simulink model "AcasXuClosedLoop_all_comp2017b"  (Simulink 9.0)
settings: Solver=VariableStepAuto  StartTime=0.0  StopTime=20  RelTol=1e-3
libraries: neural x14040, simulink x2
(part 25 of 534)

== system AcasXuClosedLoop_all_comp2017b/networks/Networks_m_6/AcasXu_1_6/Layer 5 ==
  "a{4} " : Inport  PortDimensions=50
      mask Display: e = 0.06;c1 = [120 230 180]/255;c2 = ([120 230 180]+0)/(256+128);c3 = ([120 230 180]+128)/(256+128);patch([0 1 1 0],[0 0 1 1],c1);patch([0 1 1 1-e 1-e 0+e],[0 0 1 1-e 0+e 0+e],c2);patch([0 0 1 1-e 0+e 0+e],[0 1 1 1-e 1-e 0+e],c3);plot([0 1 1 0 0],[0 0 1 1 0]);
  Delays 1 : Subsystem
      mask Display: e = 0.06;c1 = [120 150 230]/255;c2 = ([120 150 230]+0)/383;c3 = ([120 150 230]+128)/383;patch([0 1 1 0],[0 0 1 1],c1);patch([0 1 1 1-e 1-e 0+e],[0 0 1 1-e 0+e 0+e],c2);patch([0 0 1 1-e 0+e 0+e],[0 1 1 1-e 1-e 0+e],c3);plot([0 1 1 0 0],[0 0 1 1 0]);disp('0');
  "LW{5,4}" : Subsystem
      mask Display: e = 0.06;c1 = [120 150 230]/255;c2 = ([120 150 230]+0)/383;c3 = ([120 150 230]+128)/383;patch([0 1 1 0],[0 0 1 1],c1);patch([0 1 1 1-e 1-e 0+e],[0 0 1 1-e 0+e 0+e],c2);patch([0 0 1 1-e 0+e 0+e],[0 1 1 1-e 1-e 0+e],c3);plot([0 1 1 0 0],[0 0 1 1 0]);disp('W');
  "b{5}" : Constant  Value=[0.3707949999999999857180910112219862639904022216796875;-0.152619000000000004657607632907456718385219573974609375;0.048162000000000003419042826635632081888616085052490234375;0.0157031000000000010852208021105980151332914829254150390625;-0.5211959999999999926245664028101600706577301025390625;0.98311000000000003939959469789755530655384063720703125;-0.299582999999999988194332445345935411751270294189453125;-0.1225920000000000065210059574383194558322429656982421875;-0.02888759999999999938946615429813391529023647308349609375;0.281310000000000004494182803682633675634860992431640625;0.2471440000000000025703883466121624223887920379638671875;0.291130999999999973137931874589412473142147064208984375;0.12004800000000000193001170600837212987244129180908203125;-0.25239000000000000323296944770845584571361541748046875;-0.0200420000000000007867040352493859245441854000091552734375;0.06623010000000000008224532166423159651458263397216796875;0.175796000000000007812417379682301543653011322021484375;-0.12461899999999999366639968911840696819126605987548828125;0.17621100000000000651567688692011870443820953369140625;-0.450682000000000027029045668314211070537567138671875;-0.048770700000000000162714286489062942564487457275390625;0.13494200000000000638777919448330067098140716552734375;-1.5442299999999999915445414444548077881336212158203125;-0.032870799999999998630695330348316929303109645843505859375;0.153853999999999990766497148797498084604740142822265625;-0.86296600000000001084998757505672983825206756591796875;-0.5057430000000000536175548404571600258350372314453125;-0.51248400000000005061195906819193623960018157958984375;-0.269037000000000026123103680220083333551883697509765625;0.07316989999999999605417855264022364281117916107177734375;0.1014589999999999936353134444289025850594043731689453125;0.1659440000000000081659123907229513861238956451416015625;-0.63569200000000003480948862488730810582637786865234375;-0.2168150000000000077182704671940882690250873565673828125;0.158696000000000003726796649061725474894046783447265625;-1.9692700000000000759570184527547098696231842041015625;-0.76643799999999995264943208894692361354827880859375;0.299881000000000008665068662594421766698360443115234375;-0.60654200000000002557243306000600568950176239013671875;0.1070440000000000002611244553918368183076381683349609375;-0.0182207999999999988027798991652161930687725543975830078125;0.1372770000000000101270103414208278991281986236572265625;-0.08875829999999999830873065320702153258025646209716796875;0.1260400000000000131361588273648521862924098968505859375;0.07690940000000000276170197821556939743459224700927734375;0.5071440000000000392077481592423282563686370849609375;0.251952000000000009283240842705708928406238555908203125;-0.1578380000000000060733640339094563387334346771240234375;-2.0016400000000000858335624798201024532318115234375;0.03576759999999999661834948483374319039285182952880859375]
      mask Display: e = 0.06;c1 = [120 150 230]/255;c2 = ([120 150 230]+0)/383;c3 = ([120 150 230]+128)/383;patch([0 1 1 0],[0 0 1 1],c1);patch([0 1 1 1-e 1-e 0+e],[0 0 1 1-e 0+e 0+e],c2);patch([0 0 1 1-e 0+e 0+e],[0 1 1 1-e 1-e 0+e],c3);plot([0 1 1 0 0],[0 0 1 1 0]);disp('b');
  netsum : Sum  Inputs=2  SaturateOnIntegerOverflow=on  InputSameDT=on  OutDataTypeStr=Inherit: Same as first input
      mask Display: e = 0.06;c1 = [120 150 230]/255;c2 = ([120 150 230]+0)/383;c3 = ([120 150 230]+128)/383;patch([0 1 1 0],[0 0 1 1],c1);patch([0 1 1 1-e 1-e 0+e],[0 0 1 1-e 0+e 0+e],c2);patch([0 0 1 1-e 0+e 0+e],[0 1 1 1-e 1-e 0+e],c3);plot([0 1 1 0 0],[0 0 1 1 0]);disp('+');
  poslin : neural/Transfer Functions/poslin
      settings: SourceProductName=Deep Learning Toolbox  SourceProductBaseCode=NN
  "a{5}" : Outport  InitialOutput=[0;0;0;0;0;0;0;0;0;0;0;0;0;0;0;0;0;0;0;0;0;0;0;0;0;0;0;0;0;0;0;0;0;0;0;0;0;0;0;0;0;0;0;0;0;0;0;0;0;0]
      mask Display: e = 0.06;c1 = [120 230 180]/255;c2 = ([120 230 180]+0)/(256+128);c3 = ([120 230 180]+128)/(256+128);patch([0 1 1 0],[0 0 1 1],c1);patch([0 1 1 1-e 1-e 0+e],[0 0 1 1-e 0+e 0+e],c2);patch([0 0 1 1-e 0+e 0+e],[0 1 1 1-e 1-e 0+e],c3);plot([0 1 1 0 0],[0 0 1 1 0]);
  wires:
  poslin/1 -> "a{5}"/1
  netsum/1 -> poslin/1
  "b{5}"/1 -> netsum/2
  "LW{5,4}"/1("lz{5,4}") -> netsum/1
  Delays 1/1("pd{5,4}") -> "LW{5,4}"/1("ad{5,4}")
  "a{4} "/1 -> Delays 1/1("p{5}")

== system AcasXuClosedLoop_all_comp2017b/networks/Networks_m_6/AcasXu_1_6/Layer 6 ==
  "a{5} " : Inport  PortDimensions=50
      mask Display: e = 0.06;c1 = [120 230 180]/255;c2 = ([120 230 180]+0)/(256+128);c3 = ([120 230 180]+128)/(256+128);patch([0 1 1 0],[0 0 1 1],c1);patch([0 1 1 1-e 1-e 0+e],[0 0 1 1-e 0+e 0+e],c2);patch([0 0 1 1-e 0+e 0+e],[0 1 1 1-e 1-e 0+e],c3);plot([0 1 1 0 0],[0 0 1 1 0]);
  Delays 1 : Subsystem
      mask Display: e = 0.06;c1 = [120 150 230]/255;c2 = ([120 150 230]+0)/383;c3 = ([120 150 230]+128)/383;patch([0 1 1 0],[0 0 1 1],c1);patch([0 1 1 1-e 1-e 0+e],[0 0 1 1-e 0+e 0+e],c2);patch([0 0 1 1-e 0+e 0+e],[0 1 1 1-e 1-e 0+e],c3);plot([0 1 1 0 0],[0 0 1 1 0]);disp('0');
  "LW{6,5}" : Subsystem
      mask Display: e = 0.06;c1 = [120 150 230]/255;c2 = ([120 150 230]+0)/383;c3 = ([120 150 230]+128)/383;patch([0 1 1 0],[0 0 1 1],c1);patch([0 1 1 1-e 1-e 0+e],[0 0 1 1-e 0+e 0+e],c2);patch([0 0 1 1-e 0+e 0+e],[0 1 1 1-e 1-e 0+e],c3);plot([0 1 1 0 0],[0 0 1 1 0]);disp('W');
  "b{6}" : Constant  Value=[-0.09293780000000000096616048494979622773826122283935546875;-0.0196711000000000003906652779051000834442675113677978515625;-0.08280949999999999422417573669008561410009860992431640625;-0.054471199999999997398703044382273219525814056396484375;-0.03268479999999999996429522752805496565997600555419921875;0.11851000000000000422772927777259610593318939208984375;-0.13510400000000000186872739504906348884105682373046875;-0.1563849999999999962341235004714690148830413818359375;-0.660301000000000026801671992870979011058807373046875;-0.04415539999999999742907874633601750247180461883544921875;0.03159220000000000083684170704145799390971660614013671875;-0.03956199999999999994404475955889211036264896392822265625;-0.80943799999999999084110413605230860412120819091796875;0.1153389999999999970814457128653884865343570709228515625;-0.1059650000000000036326497365735122002661228179931640625;-0.39901500000000000856203996590920723974704742431640625;-0.10792300000000000503863617495881044305860996246337890625;-0.293030999999999985927701118271215818822383880615234375;0.1441389999999999893542934614742989651858806610107421875;-0.06593970000000000386553011821888503618538379669189453125;-0.9216029999999999500204239666345529258251190185546875;-0.1278370000000000061834981579522718675434589385986328125;-0.03856020000000000280682144193633575923740863800048828125;0.026769199999999999939337413934481446631252765655517578125;-0.0158453999999999990688781537073737126775085926055908203125;0.0284409000000000015961898469640800612978637218475341796875;-0.03193300000000000304911651483052992261946201324462890625;0.1266130000000000033200109328390681184828281402587890625;-0.95733100000000004303757350498926825821399688720703125;-0.335019000000000011230127938688383437693119049072265625;-0.271828000000000014058088027013582177460193634033203125;0.06131860000000000088249407781404443085193634033203125;-0.59949500000000000010658141036401502788066864013671875;0.08274660000000000348308049069601111114025115966796875;-0.280430000000000012594369991347775794565677642822265625;-0.1293509999999999937614347800263203680515289306640625;0.08134900000000000463984406451345421373844146728515625;-0.07751650000000000206856753948159166611731052398681640625;-2.159569999999999989626076057902537286281585693359375;0.046974099999999997800959050664459937252104282379150390625;0.07693919999999999925766047681463533081114292144775390625;0.07165760000000000184972037686748080886900424957275390625;-0.48705100000000001170263885796885006129741668701171875;-0.23598500000000000031974423109204508364200592041015625;-1.21154999999999990478727340814657509326934814453125;0.04766320000000000278728151670293300412595272064208984375;-0.148309999999999997388755446081631816923618316650390625;-0.9624960000000000182041048901737667620182037353515625;-0.052430699999999996752109154840582050383090972900390625;-0.9180289999999999839275233171065337955951690673828125]
      mask Display: e = 0.06;c1 = [120 150 230]/255;c2 = ([120 150 230]+0)/383;c3 = ([120 150 230]+128)/383;patch([0 1 1 0],[0 0 1 1],c1);patch([0 1 1 1-e 1-e 0+e],[0 0 1 1-e 0+e 0+e],c2);patch([0 0 1 1-e 0+e 0+e],[0 1 1 1-e 1-e 0+e],c3);plot([0 1 1 0 0],[0 0 1 1 0]);disp('b');
  netsum : Sum  Inputs=2  SaturateOnIntegerOverflow=on  InputSameDT=on  OutDataTypeStr=Inherit: Same as first input
      mask Display: e = 0.06;c1 = [120 150 230]/255;c2 = ([120 150 230]+0)/383;c3 = ([120 150 230]+128)/383;patch([0 1 1 0],[0 0 1 1],c1);patch([0 1 1 1-e 1-e 0+e],[0 0 1 1-e 0+e 0+e],c2);patch([0 0 1 1-e 0+e 0+e],[0 1 1 1-e 1-e 0+e],c3);plot([0 1 1 0 0],[0 0 1 1 0]);disp('+');
  poslin : neural/Transfer Functions/poslin
      settings: SourceProductName=Deep Learning Toolbox  SourceProductBaseCode=NN
  "a{6}" : Outport  InitialOutput=[0;0;0;0;0;0;0;0;0;0;0;0;0;0;0;0;0;0;0;0;0;0;0;0;0;0;0;0;0;0;0;0;0;0;0;0;0;0;0;0;0;0;0;0;0;0;0;0;0;0]
      mask Display: e = 0.06;c1 = [120 230 180]/255;c2 = ([120 230 180]+0)/(256+128);c3 = ([120 230 180]+128)/(256+128);patch([0 1 1 0],[0 0 1 1],c1);patch([0 1 1 1-e 1-e 0+e],[0 0 1 1-e 0+e 0+e],c2);patch([0 0 1 1-e 0+e 0+e],[0 1 1 1-e 1-e 0+e],c3);plot([0 1 1 0 0],[0 0 1 1 0]);
  wires:
  poslin/1 -> "a{6}"/1
  netsum/1 -> poslin/1
  "b{6}"/1 -> netsum/2
  "LW{6,5}"/1("lz{6,5}") -> netsum/1
  Delays 1/1("pd{6,5}") -> "LW{6,5}"/1("ad{6,5}")
  "a{5} "/1 -> Delays 1/1("p{6}")

== system AcasXuClosedLoop_all_comp2017b/networks/Networks_m_6/AcasXu_1_6/Layer 7 ==
  "a{6} " : Inport  PortDimensions=50
      mask Display: e = 0.06;c1 = [120 230 180]/255;c2 = ([120 230 180]+0)/(256+128);c3 = ([120 230 180]+128)/(256+128);patch([0 1 1 0],[0 0 1 1],c1);patch([0 1 1 1-e 1-e 0+e],[0 0 1 1-e 0+e 0+e],c2);patch([0 0 1 1-e 0+e 0+e],[0 1 1 1-e 1-e 0+e],c3);plot([0 1 1 0 0],[0 0 1 1 0]);
  Delays 1 : Subsystem
      mask Display: e = 0.06;c1 = [120 150 230]/255;c2 = ([120 150 230]+0)/383;c3 = ([120 150 230]+128)/383;patch([0 1 1 0],[0 0 1 1],c1);patch([0 1 1 1-e 1-e 0+e],[0 0 1 1-e 0+e 0+e],c2);patch([0 0 1 1-e 0+e 0+e],[0 1 1 1-e 1-e 0+e],c3);plot([0 1 1 0 0],[0 0 1 1 0]);disp('0');
  "LW{7,6}" : Subsystem
      mask Display: e = 0.06;c1 = [120 150 230]/255;c2 = ([120 150 230]+0)/383;c3 = ([120 150 230]+128)/383;patch([0 1 1 0],[0 0 1 1],c1);patch([0 1 1 1-e 1-e 0+e],[0 0 1 1-e 0+e 0+e],c2);patch([0 0 1 1-e 0+e 0+e],[0 1 1 1-e 1-e 0+e],c3);plot([0 1 1 0 0],[0 0 1 1 0]);disp('W');
  "b{7}" : Constant  Value=[-0.0201359000000000017138734875743466545827686786651611328125;-0.01936569999999999958095742158548091538250446319580078125;-0.0194450000000000004785061236134424689225852489471435546875;-0.01923740000000000172075687032702262513339519500732421875;-0.01934299999999999908339987086947076022624969482421875]
      mask Display: e = 0.06;c1 = [120 150 230]/255;c2 = ([120 150 230]+0)/383;c3 = ([120 150 230]+128)/383;patch([0 1 1 0],[0 0 1 1],c1);patch([0 1 1 1-e 1-e 0+e],[0 0 1 1-e 0+e 0+e],c2);patch([0 0 1 1-e 0+e 0+e],[0 1 1 1-e 1-e 0+e],c3);plot([0 1 1 0 0],[0 0 1 1 0]);disp('b');
  netsum : Sum  Inputs=2  SaturateOnIntegerOverflow=on  InputSameDT=on  OutDataTypeStr=Inherit: Same as first input
      mask Display: e = 0.06;c1 = [120 150 230]/255;c2 = ([120 150 230]+0)/383;c3 = ([120 150 230]+128)/383;patch([0 1 1 0],[0 0 1 1],c1);patch([0 1 1 1-e 1-e 0+e],[0 0 1 1-e 0+e 0+e],c2);patch([0 0 1 1-e 0+e 0+e],[0 1 1 1-e 1-e 0+e],c3);plot([0 1 1 0 0],[0 0 1 1 0]);disp('+');
  purelin : neural/Transfer Functions/purelin
      settings: SourceProductName=Deep Learning Toolbox
  "a{7}" : Outport  InitialOutput=[0;0;0;0;0]
      mask Display: e = 0.06;c1 = [120 230 180]/255;c2 = ([120 230 180]+0)/(256+128);c3 = ([120 230 180]+128)/(256+128);patch([0 1 1 0],[0 0 1 1],c1);patch([0 1 1 1-e 1-e 0+e],[0 0 1 1-e 0+e 0+e],c2);patch([0 0 1 1-e 0+e 0+e],[0 1 1 1-e 1-e 0+e],c3);plot([0 1 1 0 0],[0 0 1 1 0]);
  wires:
  purelin/1 -> "a{7}"/1
  netsum/1 -> purelin/1
  "b{7}"/1 -> netsum/2
  "LW{7,6}"/1("lz{7,6}") -> netsum/1
  Delays 1/1("pd{7,6}") -> "LW{7,6}"/1("ad{7,6}")
  "a{6} "/1 -> Delays 1/1("p{7}")

== system AcasXuClosedLoop_all_comp2017b/networks/Networks_m_6/AcasXu_1_6/Process Input 1 ==
  x : Inport  PortDimensions=5
      mask Display: e = 0.06;c1 = [120 230 180]/255;c2 = ([120 230 180]+0)/(256+128);c3 = ([120 230 180]+128)/(256+128);patch([0 1 1 0],[0 0 1 1],c1);patch([0 1 1 1-e 1-e 0+e],[0 0 1 1-e 0+e 0+e],c2);patch([0 0 1 1-e 0+e 0+e],[0 1 1 1-e 1-e 0+e],c3);plot([0 1 1 0 0],[0 0 1 1 0]);
  p : Outport  PortDimensions=5
      mask Display: e = 0.06;c1 = [120 230 180]/255;c2 = ([120 230 180]+0)/(256+128);c3 = ([120 230 180]+128)/(256+128);patch([0 1 1 0],[0 0 1 1],c1);patch([0 1 1 1-e 1-e 0+e],[0 0 1 1-e 0+e 0+e],c2);patch([0 0 1 1-e 0+e 0+e],[0 1 1 1-e 1-e 0+e],c3);plot([0 1 1 0 0],[0 0 1 1 0]);
  wires:
  x/1 -> p/1

== system AcasXuClosedLoop_all_comp2017b/networks/Networks_m_6/AcasXu_1_6/Process Output 1 ==
  a : Inport  PortDimensions=5
      mask Display: e = 0.06;c1 = [120 230 180]/255;c2 = ([120 230 180]+0)/(256+128);c3 = ([120 230 180]+128)/(256+128);patch([0 1 1 0],[0 0 1 1],c1);patch([0 1 1 1-e 1-e 0+e],[0 0 1 1-e 0+e 0+e],c2);patch([0 0 1 1-e 0+e 0+e],[0 1 1 1-e 1-e 0+e],c3);plot([0 1 1 0 0],[0 0 1 1 0]);
  y : Outport  PortDimensions=5
      mask Display: e = 0.06;c1 = [120 230 180]/255;c2 = ([120 230 180]+0)/(256+128);c3 = ([120 230 180]+128)/(256+128);patch([0 1 1 0],[0 0 1 1],c1);patch([0 1 1 1-e 1-e 0+e],[0 0 1 1-e 0+e 0+e],c2);patch([0 0 1 1-e 0+e 0+e],[0 1 1 1-e 1-e 0+e],c3);plot([0 1 1 0 0],[0 0 1 1 0]);
  wires:
  a/1 -> y/1

== system AcasXuClosedLoop_all_comp2017b/networks/Networks_m_6/AcasXu_2_6/Layer 1 ==
  "p{1}" : Inport  PortDimensions=5
      mask Display: e = 0.06;c1 = [120 230 180]/255;c2 = ([120 230 180]+0)/(256+128);c3 = ([120 230 180]+128)/(256+128);patch([0 1 1 0],[0 0 1 1],c1);patch([0 1 1 1-e 1-e 0+e],[0 0 1 1-e 0+e 0+e],c2);patch([0 0 1 1-e 0+e 0+e],[0 1 1 1-e 1-e 0+e],c3);plot([0 1 1 0 0],[0 0 1 1 0]);
  Delays 1 : Subsystem
      mask Display: e = 0.06;c1 = [120 150 230]/255;c2 = ([120 150 230]+0)/383;c3 = ([120 150 230]+128)/383;patch([0 1 1 0],[0 0 1 1],c1);patch([0 1 1 1-e 1-e 0+e],[0 0 1 1-e 0+e 0+e],c2);patch([0 0 1 1-e 0+e 0+e],[0 1 1 1-e 1-e 0+e],c3);plot([0 1 1 0 0],[0 0 1 1 0]);disp('0');
  "IW{1,1}" : Subsystem
      mask Display: e = 0.06;c1 = [120 150 230]/255;c2 = ([120 150 230]+0)/383;c3 = ([120 150 230]+128)/383;patch([0 1 1 0],[0 0 1 1],c1);patch([0 1 1 1-e 1-e 0+e],[0 0 1 1-e 0+e 0+e],c2);patch([0 0 1 1-e 0+e 0+e],[0 1 1 1-e 1-e 0+e],c3);plot([0 1 1 0 0],[0 0 1 1 0]);disp('W');
  "b{1}" : Constant  Value=[-0.06689219999999999888284918370118248276412487030029296875;-0.409326000000000023160140472100465558469295501708984375;0.0319440000000000001723066134218242950737476348876953125;0.01255019999999999920359261551539020729251205921173095703125;-0.459266000000000007563727422166266478598117828369140625;-0.0239383999999999985686116588112781755626201629638671875;-0.022123099999999999709654474600029061548411846160888671875;-0.377510999999999985465848340027150698006153106689453125;0.199315999999999993175237023024237714707851409912109375;0.065776200000000006884448566779610700905323028564453125;-0.25278699999999998393462874446413479745388031005859375;-0.06722430000000000072102324111256166361272335052490234375;0.52313500000000001666222715357434935867786407470703125;-0.273511000000000004117595153729780577123165130615234375;-0.112341999999999997417177155512035824358463287353515625;-0.2958819999999999783568682687473483383655548095703125;0.084172899999999994946620063274167478084564208984375;0.01048840000000000015345502646368913701735436916351318359375;-0.7274350000000000537880850970395840704441070556640625;0.03777819999999999789341842415524297393858432769775390625;0.08883770000000000555129275880972272716462612152099609375;-0.171831000000000011507239605634822510182857513427734375;-0.01849019999999999830375685405670083127915859222412109375;-0.2042090000000000016289192217300296761095523834228515625;-0.00886104000000000058268057046007015742361545562744140625;-0.398774000000000017340795466225245036184787750244140625;0.252219000000000026506796757530537433922290802001953125;0.1525330000000000019166890297128702513873577117919921875;-0.416878000000000026314950218875310383737087249755859375;0.018960600000000001064126564642720040865242481231689453125;0.340818000000000009830358749240986071527004241943359375;-0.09769619999999999693240937403970747254788875579833984375;0.031457199999999997663824302662760601378977298736572265625;-0.376325000000000020605739337042905390262603759765625;-0.1449499999999999955146989805143675766885280609130859375;-0.389840000000000019841905896100797690451145172119140625;0.016368500000000001104449864897105726413428783416748046875;-0.34057999999999999385380533567513339221477508544921875;-0.1790029999999999954507501342959585599601268768310546875;-0.0213322999999999984854781587273464538156986236572265625;0.19489699999999998691890823465655557811260223388671875;-0.07400089999999999446078646769819897599518299102783203125;-0.1605999999999999927613458794439793564379215240478515625;-0.362099000000000004195754854663391597568988800048828125;0.18424099999999998811262003073352389037609100341796875;-0.2050929999999999975290876363942516036331653594970703125;-0.0325461999999999973542941233972669579088687896728515625;-0.12970599999999998797051148358150385320186614990234375;0.04095300000000000328892468814956373535096645355224609375;-0.409160000000000023678836669205338694155216217041015625]
      mask Display: e = 0.06;c1 = [120 150 230]/255;c2 = ([120 150 230]+0)/383;c3 = ([120 150 230]+128)/383;patch([0 1 1 0],[0 0 1 1],c1);patch([0 1 1 1-e 1-e 0+e],[0 0 1 1-e 0+e 0+e],c2);patch([0 0 1 1-e 0+e 0+e],[0 1 1 1-e 1-e 0+e],c3);plot([0 1 1 0 0],[0 0 1 1 0]);disp('b');
  netsum : Sum  Inputs=2  SaturateOnIntegerOverflow=on  InputSameDT=on  OutDataTypeStr=Inherit: Same as first input
      mask Display: e = 0.06;c1 = [120 150 230]/255;c2 = ([120 150 230]+0)/383;c3 = ([120 150 230]+128)/383;patch([0 1 1 0],[0 0 1 1],c1);patch([0 1 1 1-e 1-e 0+e],[0 0 1 1-e 0+e 0+e],c2);patch([0 0 1 1-e 0+e 0+e],[0 1 1 1-e 1-e 0+e],c3);plot([0 1 1 0 0],[0 0 1 1 0]);disp('+');
  poslin : neural/Transfer Functions/poslin
      settings: SourceProductName=Deep Learning Toolbox  SourceProductBaseCode=NN
  "a{1}" : Outport  InitialOutput=[0;0;0;0;0;0;0;0;0;0;0;0;0;0;0;0;0;0;0;0;0;0;0;0;0;0;0;0;0;0;0;0;0;0;0;0;0;0;0;0;0;0;0;0;0;0;0;0;0;0]
      mask Display: e = 0.06;c1 = [120 230 180]/255;c2 = ([120 230 180]+0)/(256+128);c3 = ([120 230 180]+128)/(256+128);patch([0 1 1 0],[0 0 1 1],c1);patch([0 1 1 1-e 1-e 0+e],[0 0 1 1-e 0+e 0+e],c2);patch([0 0 1 1-e 0+e 0+e],[0 1 1 1-e 1-e 0+e],c3);plot([0 1 1 0 0],[0 0 1 1 0]);
  wires:
  poslin/1 -> "a{1}"/1
  netsum/1 -> poslin/1
  "b{1}"/1 -> netsum/2
  "IW{1,1}"/1("iz{1,1}") -> netsum/1
  Delays 1/1("pd{1,1}") -> "IW{1,1}"/1("pd{1,1}")
  "p{1}"/1 -> Delays 1/1("p{1}")

== system AcasXuClosedLoop_all_comp2017b/networks/Networks_m_6/AcasXu_2_6/Layer 2 ==
  "a{1} " : Inport  PortDimensions=50
      mask Display: e = 0.06;c1 = [120 230 180]/255;c2 = ([120 230 180]+0)/(256+128);c3 = ([120 230 180]+128)/(256+128);patch([0 1 1 0],[0 0 1 1],c1);patch([0 1 1 1-e 1-e 0+e],[0 0 1 1-e 0+e 0+e],c2);patch([0 0 1 1-e 0+e 0+e],[0 1 1 1-e 1-e 0+e],c3);plot([0 1 1 0 0],[0 0 1 1 0]);
  Delays 1 : Subsystem
      mask Display: e = 0.06;c1 = [120 150 230]/255;c2 = ([120 150 230]+0)/383;c3 = ([120 150 230]+128)/383;patch([0 1 1 0],[0 0 1 1],c1);patch([0 1 1 1-e 1-e 0+e],[0 0 1 1-e 0+e 0+e],c2);patch([0 0 1 1-e 0+e 0+e],[0 1 1 1-e 1-e 0+e],c3);plot([0 1 1 0 0],[0 0 1 1 0]);disp('0');
  "LW{2,1}" : Subsystem
      mask Display: e = 0.06;c1 = [120 150 230]/255;c2 = ([120 150 230]+0)/383;c3 = ([120 150 230]+128)/383;patch([0 1 1 0],[0 0 1 1],c1);patch([0 1 1 1-e 1-e 0+e],[0 0 1 1-e 0+e 0+e],c2);patch([0 0 1 1-e 0+e 0+e],[0 1 1 1-e 1-e 0+e],c3);plot([0 1 1 0 0],[0 0 1 1 0]);disp('W');
  "b{2}" : Constant  Value=[-0.0267394999999999993800514630493125878274440765380859375;1.202420000000000044337866711430251598358154296875;0.0700510000000000021547208461925038136541843414306640625;-0.00306441999999999999115818383188525331206619739532470703125;1.192660000000000053432813729159533977508544921875;-1.0100899999999999323563315556384623050689697265625;-0.8294289999999999718482968091848306357860565185546875;-0.032258399999999999574473719121670001186430454254150390625;-0.3208860000000000045616843635798431932926177978515625;0.88836499999999996024513393422239460051059722900390625;-0.0128079000000000005343725462125803460367023944854736328125;-0.57480799999999998561861502821557223796844482421875;-0.015656599999999999683897300428725429810583591461181640625;0.382274999999999975930364826126606203615665435791015625;1.26560000000000005826450433232821524143218994140625;-0.341955000000000008952838470577262341976165771484375;-0.06256899999999999961719510110924602486193180084228515625;-1.0951500000000000678568312650895677506923675537109375;1.0387100000000000221689333557151257991790771484375;0.6711549999999999460698063558083958923816680908203125;0.032979899999999999493649482928958605043590068817138671875;-0.265936000000000005716316309190005995333194732666015625;-1.6442200000000000148503431773860938847064971923828125;0.405764999999999986801668683256139047443866729736328125;-0.015960900000000000087396756498492322862148284912109375;0.4590520000000000155893076225765980780124664306640625;1.266480000000000050164317144663073122501373291015625;-0.006008389999999999737945177713527300511486828327178955078125;0.276619000000000003769429213207331486046314239501953125;0.12648300000000001208633193527930416166782379150390625;0.28959400000000001806910177037934772670269012451171875;0.92441099999999998271249523895676247775554656982421875;-0.020860099999999999476063550218896125443279743194580078125;0.45288600000000001077893330148071981966495513916015625;0.371752000000000026869173552768188528716564178466796875;-0.03343390000000000250590659334193333052098751068115234375;0.13280300000000000437694325228221714496612548828125;0.22576099999999998946265122867771424353122711181640625;0.88422199999999995245758555029169656336307525634765625;-0.274563999999999974743758457407238893210887908935546875;-0.31437199999999998478728002737625502049922943115234375;-0.343855000000000021742607714259065687656402587890625;0.01097919999999999972339903564488849951885640621185302734375;-0.14926600000000000978417347141657955944538116455078125;0.238543000000000005034195282860309816896915435791015625;-0.11582599999999999840216702295947470702230930328369140625;-0.98861600000000005028510940974229015409946441650390625;-0.12259100000000000552091705685597844421863555908203125;-0.6149240000000000261337618212564848363399505615234375;-0.031387400000000002797673204213424469344317913055419921875]
      mask Display: e = 0.06;c1 = [120 150 230]/255;c2 = ([120 150 230]+0)/383;c3 = ([120 150 230]+128)/383;patch([0 1 1 0],[0 0 1 1],c1);patch([0 1 1 1-e 1-e 0+e],[0 0 1 1-e 0+e 0+e],c2);patch([0 0 1 1-e 0+e 0+e],[0 1 1 1-e 1-e 0+e],c3);plot([0 1 1 0 0],[0 0 1 1 0]);disp('b');
  netsum : Sum  Inputs=2  SaturateOnIntegerOverflow=on  InputSameDT=on  OutDataTypeStr=Inherit: Same as first input
      mask Display: e = 0.06;c1 = [120 150 230]/255;c2 = ([120 150 230]+0)/383;c3 = ([120 150 230]+128)/383;patch([0 1 1 0],[0 0 1 1],c1);patch([0 1 1 1-e 1-e 0+e],[0 0 1 1-e 0+e 0+e],c2);patch([0 0 1 1-e 0+e 0+e],[0 1 1 1-e 1-e 0+e],c3);plot([0 1 1 0 0],[0 0 1 1 0]);disp('+');
  poslin : neural/Transfer Functions/poslin
      settings: SourceProductName=Deep Learning Toolbox  SourceProductBaseCode=NN
  "a{2}" : Outport  InitialOutput=[0;0;0;0;0;0;0;0;0;0;0;0;0;0;0;0;0;0;0;0;0;0;0;0;0;0;0;0;0;0;0;0;0;0;0;0;0;0;0;0;0;0;0;0;0;0;0;0;0;0]
      mask Display: e = 0.06;c1 = [120 230 180]/255;c2 = ([120 230 180]+0)/(256+128);c3 = ([120 230 180]+128)/(256+128);patch([0 1 1 0],[0 0 1 1],c1);patch([0 1 1 1-e 1-e 0+e],[0 0 1 1-e 0+e 0+e],c2);patch([0 0 1 1-e 0+e 0+e],[0 1 1 1-e 1-e 0+e],c3);plot([0 1 1 0 0],[0 0 1 1 0]);
  wires:
  poslin/1 -> "a{2}"/1
  netsum/1 -> poslin/1
  "b{2}"/1 -> netsum/2
  "LW{2,1}"/1("lz{2,1}") -> netsum/1
  Delays 1/1("pd{2,1}") -> "LW{2,1}"/1("ad{2,1}")
  "a{1} "/1 -> Delays 1/1("p{2}")

== system AcasXuClosedLoop_all_comp2017b/networks/Networks_m_6/AcasXu_2_6/Layer 3 ==
  "a{2} " : Inport  PortDimensions=50
      mask Display: e = 0.06;c1 = [120 230 180]/255;c2 = ([120 230 180]+0)/(256+128);c3 = ([120 230 180]+128)/(256+128);patch([0 1 1 0],[0 0 1 1],c1);patch([0 1 1 1-e 1-e 0+e],[0 0 1 1-e 0+e 0+e],c2);patch([0 0 1 1-e 0+e 0+e],[0 1 1 1-e 1-e 0+e],c3);plot([0 1 1 0 0],[0 0 1 1 0]);
  Delays 1 : Subsystem
      mask Display: e = 0.06;c1 = [120 150 230]/255;c2 = ([120 150 230]+0)/383;c3 = ([120 150 230]+128)/383;patch([0 1 1 0],[0 0 1 1],c1);patch([0 1 1 1-e 1-e 0+e],[0 0 1 1-e 0+e 0+e],c2);patch([0 0 1 1-e 0+e 0+e],[0 1 1 1-e 1-e 0+e],c3);plot([0 1 1 0 0],[0 0 1 1 0]);disp('0');
  "LW{3,2}" : Subsystem
      mask Display: e = 0.06;c1 = [120 150 230]/255;c2 = ([120 150 230]+0)/383;c3 = ([120 150 230]+128)/383;patch([0 1 1 0],[0 0 1 1],c1);patch([0 1 1 1-e 1-e 0+e],[0 0 1 1-e 0+e 0+e],c2);patch([0 0 1 1-e 0+e 0+e],[0 1 1 1-e 1-e 0+e],c3);plot([0 1 1 0 0],[0 0 1 1 0]);disp('W');
  "b{3}" : Constant  Value=[0.337579000000000017944756791621330194175243377685546875;0.1758309999999999873043776688064099289476871490478515625;-0.89985499999999996045829675495042465627193450927734375;-1.355259999999999909192638369859196245670318603515625;0.63707100000000005390887736211880110204219818115234375;-1.4238299999999999290167806975659914314746856689453125;1.067299999999999915445414444548077881336212158203125;-0.7084740000000000481605866298195905983448028564453125;-1.4174700000000000077449158197850920259952545166015625;-0.11779499999999999693134355993606732226908206939697265625;1.2623400000000000176214598468504846096038818359375;0.51268299999999999982946974341757595539093017578125;-2.148000000000000131450406115618534386157989501953125;0.312717999999999995974775401919032447040081024169921875;-0.048067300000000000304023473063352867029607295989990234375;-0.87573500000000004117595153729780577123165130615234375;-0.2186829999999999885051948922409792430698871612548828125;0.3040789999999999881907797316671349108219146728515625;-0.01367649999999999942124073726290589547716081142425537109375;0.54978899999999997216804104027687571942806243896484375;0.33024799999999998600941353288362734019756317138671875;0.0674445999999999934448879912451957352459430694580078125;-2.195530000000000203641548068844713270664215087890625;-0.51946400000000003682742999444599263370037078857421875;-0.2409900000000000097610808325043763034045696258544921875;-1.511249999999999982236431605997495353221893310546875;-0.310750000000000026201263381153694353997707366943359375;-0.1705149999999999999023003738329862244427204132080078125;0.1558479999999999865423205847037024796009063720703125;-0.92239499999999996493471599023905582726001739501953125;-0.00433562000000000009880540829954043147154152393341064453125;0.0231082999999999982865261927145184017717838287353515625;0.090483499999999994489741084180423058569431304931640625;-1.0950200000000001043787278831587173044681549072265625;0.0511633999999999977692510810811654664576053619384765625;-0.0700029000000000067860383978768368251621723175048828125;-1.29147999999999996134647517465054988861083984375;-0.0060363099999999995926014406677495571784675121307373046875;0.06676559999999999439612707874402985908091068267822265625;0.52939400000000003121414238194120116531848907470703125;0.294132999999999977802644934854470193386077880859375;1.6080300000000000704147851138259284198284149169921875;-0.0911335000000000061692873032370698638260364532470703125;-1.5363599999999999479172174687846563756465911865234375;-2.32176999999999988943955031572841107845306396484375;-0.2760409999999999808295569891924969851970672607421875;-0.085157399999999994211208331762463785707950592041015625;-0.08978930000000000244408937533080461435019969940185546875;0.59859600000000001696065510259359143674373626708984375;0.1879679999999999961968200068440637551248073577880859375]
      mask Display: e = 0.06;c1 = [120 150 230]/255;c2 = ([120 150 230]+0)/383;c3 = ([120 150 230]+128)/383;patch([0 1 1 0],[0 0 1 1],c1);patch([0 1 1 1-e 1-e 0+e],[0 0 1 1-e 0+e 0+e],c2);patch([0 0 1 1-e 0+e 0+e],[0 1 1 1-e 1-e 0+e],c3);plot([0 1 1 0 0],[0 0 1 1 0]);disp('b');
  netsum : Sum  Inputs=2  SaturateOnIntegerOverflow=on  InputSameDT=on  OutDataTypeStr=Inherit: Same as first input
      mask Display: e = 0.06;c1 = [120 150 230]/255;c2 = ([120 150 230]+0)/383;c3 = ([120 150 230]+128)/383;patch([0 1 1 0],[0 0 1 1],c1);patch([0 1 1 1-e 1-e 0+e],[0 0 1 1-e 0+e 0+e],c2);patch([0 0 1 1-e 0+e 0+e],[0 1 1 1-e 1-e 0+e],c3);plot([0 1 1 0 0],[0 0 1 1 0]);disp('+');
  poslin : neural/Transfer Functions/poslin
      settings: SourceProductName=Deep Learning Toolbox  SourceProductBaseCode=NN
  "a{3}" : Outport  InitialOutput=[0;0;0;0;0;0;0;0;0;0;0;0;0;0;0;0;0;0;0;0;0;0;0;0;0;0;0;0;0;0;0;0;0;0;0;0;0;0;0;0;0;0;0;0;0;0;0;0;0;0]
      mask Display: e = 0.06;c1 = [120 230 180]/255;c2 = ([120 230 180]+0)/(256+128);c3 = ([120 230 180]+128)/(256+128);patch([0 1 1 0],[0 0 1 1],c1);patch([0 1 1 1-e 1-e 0+e],[0 0 1 1-e 0+e 0+e],c2);patch([0 0 1 1-e 0+e 0+e],[0 1 1 1-e 1-e 0+e],c3);plot([0 1 1 0 0],[0 0 1 1 0]);
  wires:
  poslin/1 -> "a{3}"/1
  netsum/1 -> poslin/1
  "b{3}"/1 -> netsum/2
  "LW{3,2}"/1("lz{3,2}") -> netsum/1
  Delays 1/1("pd{3,2}") -> "LW{3,2}"/1("ad{3,2}")
  "a{2} "/1 -> Delays 1/1("p{3}")

== system AcasXuClosedLoop_all_comp2017b/networks/Networks_m_6/AcasXu_2_6/Layer 4 ==
  "a{3} " : Inport  PortDimensions=50
      mask Display: e = 0.06;c1 = [120 230 180]/255;c2 = ([120 230 180]+0)/(256+128);c3 = ([120 230 180]+128)/(256+128);patch([0 1 1 0],[0 0 1 1],c1);patch([0 1 1 1-e 1-e 0+e],[0 0 1 1-e 0+e 0+e],c2);patch([0 0 1 1-e 0+e 0+e],[0 1 1 1-e 1-e 0+e],c3);plot([0 1 1 0 0],[0 0 1 1 0]);
  Delays 1 : Subsystem
      mask Display: e = 0.06;c1 = [120 150 230]/255;c2 = ([120 150 230]+0)/383;c3 = ([120 150 230]+128)/383;patch([0 1 1 0],[0 0 1 1],c1);patch([0 1 1 1-e 1-e 0+e],[0 0 1 1-e 0+e 0+e],c2);patch([0 0 1 1-e 0+e 0+e],[0 1 1 1-e 1-e 0+e],c3);plot([0 1 1 0 0],[0 0 1 1 0]);disp('0');
  "LW{4,3}" : Subsystem
      mask Display: e = 0.06;c1 = [120 150 230]/255;c2 = ([120 150 230]+0)/383;c3 = ([120 150 230]+128)/383;patch([0 1 1 0],[0 0 1 1],c1);patch([0 1 1 1-e 1-e 0+e],[0 0 1 1-e 0+e 0+e],c2);patch([0 0 1 1-e 0+e 0+e],[0 1 1 1-e 1-e 0+e],c3);plot([0 1 1 0 0],[0 0 1 1 0]);disp('W');
  "b{4}" : Constant  Value=[-1.8131999999999999229061131700291298329830169677734375;-1.32214000000000009293898983742110431194305419921875;0.022890000000000000623945339839337975718080997467041015625;-1.61071000000000008611777957412414252758026123046875;1.3999900000000000677147227179375477135181427001953125;-1.4489099999999999202060507741407491266727447509765625;-0.684255999999999975358377923839725553989410400390625;1.2336300000000000043343106881366111338138580322265625;0.2019700000000000106314956838104990310966968536376953125;-0.84199999999999997069011214989586733281612396240234375;2.57085000000000007958078640513122081756591796875;-0.203320000000000000728306304154102690517902374267578125;-0.8889810000000000211883843803661875426769256591796875;-0.48887599999999997724131617360399104654788970947265625;-0.9993790000000000173230318978312425315380096435546875;1.00560999999999989285015544737689197063446044921875;-2.349089999999999900381908446433953940868377685546875;1.941240000000000076596506914938800036907196044921875;1.97008999999999989682919476763345301151275634765625;-0.95770900000000003249311930630938149988651275634765625;-0.35629100000000002435029955449863336980342864990234375;-0.057073199999999997544808394422943820245563983917236328125;-0.72231100000000003635847178884432651102542877197265625;0.04238150000000000250377496513465302996337413787841796875;-0.0196524999999999998301358772323510493151843547821044921875;0.61761399999999999632649405612028203904628753662109375;-0.292733999999999994212629417233983986079692840576171875;1.06878999999999990677679306827485561370849609375;-1.3587000000000000188293824976426549255847930908203125;-3.359609999999999985220711096189916133880615234375;-1.8428100000000000591171556152403354644775390625;-0.95105899999999998772892695342306979000568389892578125;0.2166579999999999894821911539111169986426830291748046875;-1.536259999999999958930629873066209256649017333984375;1.70056999999999991501908880309201776981353759765625;-0.99028799999999994607691178316599689424037933349609375;-3.237049999999999982946974341757595539093017578125;-1.221719999999999917150717010372318327426910400390625;0.203095999999999998752997498741024173796176910400390625;-0.50183400000000000229505303650512360036373138427734375;0.52678700000000000525091081726714037358760833740234375;0.52920500000000003648636948128114454448223114013671875;0.315236000000000016196821661651483736932277679443359375;-0.334826000000000012501999435698962770402431488037109375;-2.152320000000000010942358130705542862415313720703125;2.370200000000000084554585555451922118663787841796875;0.52536799999999994614796605674200691282749176025390625;-0.1039009999999999933617544911612640134990215301513671875;0.25243999999999999772626324556767940521240234375;-0.026639900000000001079047962093682144768536090850830078125]
      mask Display: e = 0.06;c1 = [120 150 230]/255;c2 = ([120 150 230]+0)/383;c3 = ([120 150 230]+128)/383;patch([0 1 1 0],[0 0 1 1],c1);patch([0 1 1 1-e 1-e 0+e],[0 0 1 1-e 0+e 0+e],c2);patch([0 0 1 1-e 0+e 0+e],[0 1 1 1-e 1-e 0+e],c3);plot([0 1 1 0 0],[0 0 1 1 0]);disp('b');
  netsum : Sum  Inputs=2  SaturateOnIntegerOverflow=on  InputSameDT=on  OutDataTypeStr=Inherit: Same as first input
      mask Display: e = 0.06;c1 = [120 150 230]/255;c2 = ([120 150 230]+0)/383;c3 = ([120 150 230]+128)/383;patch([0 1 1 0],[0 0 1 1],c1);patch([0 1 1 1-e 1-e 0+e],[0 0 1 1-e 0+e 0+e],c2);patch([0 0 1 1-e 0+e 0+e],[0 1 1 1-e 1-e 0+e],c3);plot([0 1 1 0 0],[0 0 1 1 0]);disp('+');
  poslin : neural/Transfer Functions/poslin
      settings: SourceProductName=Deep Learning Toolbox  SourceProductBaseCode=NN
  "a{4}" : Outport  InitialOutput=[0;0;0;0;0;0;0;0;0;0;0;0;0;0;0;0;0;0;0;0;0;0;0;0;0;0;0;0;0;0;0;0;0;0;0;0;0;0;0;0;0;0;0;0;0;0;0;0;0;0]
      mask Display: e = 0.06;c1 = [120 230 180]/255;c2 = ([120 230 180]+0)/(256+128);c3 = ([120 230 180]+128)/(256+128);patch([0 1 1 0],[0 0 1 1],c1);patch([0 1 1 1-e 1-e 0+e],[0 0 1 1-e 0+e 0+e],c2);patch([0 0 1 1-e 0+e 0+e],[0 1 1 1-e 1-e 0+e],c3);plot([0 1 1 0 0],[0 0 1 1 0]);
  wires:
  poslin/1 -> "a{4}"/1
  netsum/1 -> poslin/1
  "b{4}"/1 -> netsum/2
  "LW{4,3}"/1("lz{4,3}") -> netsum/1
  Delays 1/1("pd{4,3}") -> "LW{4,3}"/1("ad{4,3}")
  "a{3} "/1 -> Delays 1/1("p{4}")

== system AcasXuClosedLoop_all_comp2017b/networks/Networks_m_6/AcasXu_2_6/Layer 5 ==
  "a{4} " : Inport  PortDimensions=50
      mask Display: e = 0.06;c1 = [120 230 180]/255;c2 = ([120 230 180]+0)/(256+128);c3 = ([120 230 180]+128)/(256+128);patch([0 1 1 0],[0 0 1 1],c1);patch([0 1 1 1-e 1-e 0+e],[0 0 1 1-e 0+e 0+e],c2);patch([0 0 1 1-e 0+e 0+e],[0 1 1 1-e 1-e 0+e],c3);plot([0 1 1 0 0],[0 0 1 1 0]);
  Delays 1 : Subsystem
      mask Display: e = 0.06;c1 = [120 150 230]/255;c2 = ([120 150 230]+0)/383;c3 = ([120 150 230]+128)/383;patch([0 1 1 0],[0 0 1 1],c1);patch([0 1 1 1-e 1-e 0+e],[0 0 1 1-e 0+e 0+e],c2);patch([0 0 1 1-e 0+e 0+e],[0 1 1 1-e 1-e 0+e],c3);plot([0 1 1 0 0],[0 0 1 1 0]);disp('0');
  "LW{5,4}" : Subsystem
      mask Display: e = 0.06;c1 = [120 150 230]/255;c2 = ([120 150 230]+0)/383;c3 = ([120 150 230]+128)/383;patch([0 1 1 0],[0 0 1 1],c1);patch([0 1 1 1-e 1-e 0+e],[0 0 1 1-e 0+e 0+e],c2);patch([0 0 1 1-e 0+e 0+e],[0 1 1 1-e 1-e 0+e],c3);plot([0 1 1 0 0],[0 0 1 1 0]);disp('W');
  "b{5}" : Constant  Value=[1.9579500000000000792255150372511707246303558349609375;-0.3932430000000000092086338554508984088897705078125;-0.031332199999999997552802000200244947336614131927490234375;-1.06177000000000010260237104375846683979034423828125;-0.006886759999999999583286669491144493804313242435455322265625;-0.052531500000000001804334459620804409496486186981201171875;-0.316556999999999977291054165107198059558868408203125;0.84502200000000005086775445306557230651378631591796875;3.521860000000000212594386539421975612640380859375;-4.819069999999999964757080306299030780792236328125;2.24476999999999993207211446133442223072052001953125;-4.23242000000000029302782422746531665325164794921875;-1.58119999999999993889332472463138401508331298828125;-0.730088999999999987977616910939104855060577392578125;1.052929999999999921556081972084939479827880859375;2.82176999999999988943955031572841107845306396484375;2.145830000000000126192389870993793010711669921875;2.639600000000000168398628375143744051456451416015625;-0.8551450000000000439825953435502015054225921630859375;-0.06845790000000000208313366556467371992766857147216796875;0.2912069999999999936335370875895023345947265625;0.304570999999999980634157736858469434082508087158203125;-0.7341729999999999645154957761405967175960540771484375;1.543260000000000076170181273482739925384521484375;1.3247400000000000286348722511320374906063079833984375;-0.87105999999999994543031789362430572509765625;0.6899800000000000377298192688613198697566986083984375;1.503390000000000004121147867408581078052520751953125;0.742720999999999964558128340286202728748321533203125;1.955810000000000048459014578838832676410675048828125;-0.68417300000000003112887725364998914301395416259765625;0.073559399999999997010746710657258518040180206298828125;-1.4527200000000000112976294985855929553508758544921875;2.880879999999999885318402448319830000400543212890625;-0.249736000000000013532286402551108039915561676025390625;-0.60643299999999999982946974341757595539093017578125;-0.032684499999999998276489776571906986646354198455810546875;0.89280400000000004201439196549472399055957794189453125;-0.14602999999999999314326259991503320634365081787109375;-0.0107662000000000000754507567535256384871900081634521484375;-1.86671999999999993491428540437482297420501708984375;-2.539660000000000028563817977556027472019195556640625;-0.41064800000000001301003749176743440330028533935546875;4.34902000000000032997604648699052631855010986328125;2.95758000000000009777068044058978557586669921875;-1.0590699999999999558752961092977784574031829833984375;-2.93874000000000012988721209694631397724151611328125;2.458810000000000162145852300454862415790557861328125;-0.9789229999999999876081346883438527584075927734375;-1.71516000000000001790567694115452468395233154296875]
      mask Display: e = 0.06;c1 = [120 150 230]/255;c2 = ([120 150 230]+0)/383;c3 = ([120 150 230]+128)/383;patch([0 1 1 0],[0 0 1 1],c1);patch([0 1 1 1-e 1-e 0+e],[0 0 1 1-e 0+e 0+e],c2);patch([0 0 1 1-e 0+e 0+e],[0 1 1 1-e 1-e 0+e],c3);plot([0 1 1 0 0],[0 0 1 1 0]);disp('b');
  netsum : Sum  Inputs=2  SaturateOnIntegerOverflow=on  InputSameDT=on  OutDataTypeStr=Inherit: Same as first input
      mask Display: e = 0.06;c1 = [120 150 230]/255;c2 = ([120 150 230]+0)/383;c3 = ([120 150 230]+128)/383;patch([0 1 1 0],[0 0 1 1],c1);patch([0 1 1 1-e 1-e 0+e],[0 0 1 1-e 0+e 0+e],c2);patch([0 0 1 1-e 0+e 0+e],[0 1 1 1-e 1-e 0+e],c3);plot([0 1 1 0 0],[0 0 1 1 0]);disp('+');
  poslin : neural/Transfer Functions/poslin
      settings: SourceProductName=Deep Learning Toolbox  SourceProductBaseCode=NN
  "a{5}" : Outport  InitialOutput=[0;0;0;0;0;0;0;0;0;0;0;0;0;0;0;0;0;0;0;0;0;0;0;0;0;0;0;0;0;0;0;0;0;0;0;0;0;0;0;0;0;0;0;0;0;0;0;0;0;0]
      mask Display: e = 0.06;c1 = [120 230 180]/255;c2 = ([120 230 180]+0)/(256+128);c3 = ([120 230 180]+128)/(256+128);patch([0 1 1 0],[0 0 1 1],c1);patch([0 1 1 1-e 1-e 0+e],[0 0 1 1-e 0+e 0+e],c2);patch([0 0 1 1-e 0+e 0+e],[0 1 1 1-e 1-e 0+e],c3);plot([0 1 1 0 0],[0 0 1 1 0]);
  wires:
  poslin/1 -> "a{5}"/1
  netsum/1 -> poslin/1
  "b{5}"/1 -> netsum/2
  "LW{5,4}"/1("lz{5,4}") -> netsum/1
  Delays 1/1("pd{5,4}") -> "LW{5,4}"/1("ad{5,4}")
  "a{4} "/1 -> Delays 1/1("p{5}")

== system AcasXuClosedLoop_all_comp2017b/networks/Networks_m_6/AcasXu_2_6/Layer 6 ==
  "a{5} " : Inport  PortDimensions=50
      mask Display: e = 0.06;c1 = [120 230 180]/255;c2 = ([120 230 180]+0)/(256+128);c3 = ([120 230 180]+128)/(256+128);patch([0 1 1 0],[0 0 1 1],c1);patch([0 1 1 1-e 1-e 0+e],[0 0 1 1-e 0+e 0+e],c2);patch([0 0 1 1-e 0+e 0+e],[0 1 1 1-e 1-e 0+e],c3);plot([0 1 1 0 0],[0 0 1 1 0]);
  Delays 1 : Subsystem
      mask Display: e = 0.06;c1 = [120 150 230]/255;c2 = ([120 150 230]+0)/383;c3 = ([120 150 230]+128)/383;patch([0 1 1 0],[0 0 1 1],c1);patch([0 1 1 1-e 1-e 0+e],[0 0 1 1-e 0+e 0+e],c2);patch([0 0 1 1-e 0+e 0+e],[0 1 1 1-e 1-e 0+e],c3);plot([0 1 1 0 0],[0 0 1 1 0]);disp('0');
  "LW{6,5}" : Subsystem
      mask Display: e = 0.06;c1 = [120 150 230]/255;c2 = ([120 150 230]+0)/383;c3 = ([120 150 230]+128)/383;patch([0 1 1 0],[0 0 1 1],c1);patch([0 1 1 1-e 1-e 0+e],[0 0 1 1-e 0+e 0+e],c2);patch([0 0 1 1-e 0+e 0+e],[0 1 1 1-e 1-e 0+e],c3);plot([0 1 1 0 0],[0 0 1 1 0]);disp('W');
  "b{6}" : Constant  Value=[-3.22956000000000020833113012486137449741363525390625;0.349115000000000008650857807879219762980937957763671875;-2.393730000000000135429445435875095427036285400390625;-3.941739999999999799484839968499727547168731689453125;4.24969999999999981099563228781335055828094482421875;-5.149029999999999773763192933984100818634033203125;-1.0581899999999999639754832969629205763339996337890625;-1.5185800000000000409272615797817707061767578125;-2.38117000000000000881072992342524230480194091796875;1.1363099999999999312194631784223020076751708984375;1.2455600000000000004973799150320701301097869873046875;1.375879999999999991899812812334857881069183349609375;1.538650000000000073185901783290319144725799560546875;0.83673100000000000253663756666355766355991363525390625;-1.1716699999999999892708046900224871933460235595703125;-0.0281448000000000010667466909808354103006422519683837890625;0.332537000000000027011282099920208565890789031982421875;2.0692099999999999937472239253111183643341064453125;-0.60126500000000004941824727211496792733669281005859375;-1.56346000000000007190692485892213881015777587890625;-0.598551000000000055223381423274986445903778076171875;0.5765599999999999614175294482265599071979522705078125;0.430995999999999990226484669619821943342685699462890625;-3.1732800000000001006128513836301863193511962890625;-0.7407179999999999875370804147678427398204803466796875;-1.074899999999999966604491419275291264057159423828125;-3.631209999999999826769681021687574684619903564453125;-3.93496999999999985675458447076380252838134765625;4.42203999999999997072563928668387234210968017578125;0.2724940000000000139834810397587716579437255859375;-3.026149999999999895550217843265272676944732666015625;1.0248299999999999077004986247629858553409576416015625;0.1357960000000000000408562073062057606875896453857421875;-0.1536589999999999900381908446433953940868377685546875;-1.15355000000000007531752999057061970233917236328125;0.291416999999999981607601284849806688725948333740234375;0.41911100000000001131184035330079495906829833984375;-2.28042999999999995708321876008994877338409423828125;0.36378500000000002501110429875552654266357421875;-0.7394279999999999741788769824779592454433441162109375;1.0599600000000000132871491587138734757900238037109375;1.020119999999999915729631538852117955684661865234375;-4.5591699999999999448618837050162255764007568359375;0.35124699999999997590549583037500269711017608642578125;0.492246000000000016871837260623578913509845733642578125;-3.487950000000000105870867628254927694797515869140625;-3.2962199999999999278088580467738211154937744140625;-0.1980590000000000128199673099516076035797595977783203125;0.68778700000000003722533392647164873778820037841796875;5.439479999999999648707671440206468105316162109375]
      mask Display: e = 0.06;c1 = [120 150 230]/255;c2 = ([120 150 230]+0)/383;c3 = ([120 150 230]+128)/383;patch([0 1 1 0],[0 0 1 1],c1);patch([0 1 1 1-e 1-e 0+e],[0 0 1 1-e 0+e 0+e],c2);patch([0 0 1 1-e 0+e 0+e],[0 1 1 1-e 1-e 0+e],c3);plot([0 1 1 0 0],[0 0 1 1 0]);disp('b');
  netsum : Sum  Inputs=2  SaturateOnIntegerOverflow=on  InputSameDT=on  OutDataTypeStr=Inherit: Same as first input
      mask Display: e = 0.06;c1 = [120 150 230]/255;c2 = ([120 150 230]+0)/383;c3 = ([120 150 230]+128)/383;patch([0 1 1 0],[0 0 1 1],c1);patch([0 1 1 1-e 1-e 0+e],[0 0 1 1-e 0+e 0+e],c2);patch([0 0 1 1-e 0+e 0+e],[0 1 1 1-e 1-e 0+e],c3);plot([0 1 1 0 0],[0 0 1 1 0]);disp('+');
  poslin : neural/Transfer Functions/poslin
      settings: SourceProductName=Deep Learning Toolbox  SourceProductBaseCode=NN
  "a{6}" : Outport  InitialOutput=[0;0;0;0;0;0;0;0;0;0;0;0;0;0;0;0;0;0;0;0;0;0;0;0;0;0;0;0;0;0;0;0;0;0;0;0;0;0;0;0;0;0;0;0;0;0;0;0;0;0]
      mask Display: e = 0.06;c1 = [120 230 180]/255;c2 = ([120 230 180]+0)/(256+128);c3 = ([120 230 180]+128)/(256+128);patch([0 1 1 0],[0 0 1 1],c1);patch([0 1 1 1-e 1-e 0+e],[0 0 1 1-e 0+e 0+e],c2);patch([0 0 1 1-e 0+e 0+e],[0 1 1 1-e 1-e 0+e],c3);plot([0 1 1 0 0],[0 0 1 1 0]);
  wires:
  poslin/1 -> "a{6}"/1
  netsum/1 -> poslin/1
  "b{6}"/1 -> netsum/2
  "LW{6,5}"/1("lz{6,5}") -> netsum/1
  Delays 1/1("pd{6,5}") -> "LW{6,5}"/1("ad{6,5}")
  "a{5} "/1 -> Delays 1/1("p{6}")

== system AcasXuClosedLoop_all_comp2017b/networks/Networks_m_6/AcasXu_2_6/Layer 7 ==
  "a{6} " : Inport  PortDimensions=50
      mask Display: e = 0.06;c1 = [120 230 180]/255;c2 = ([120 230 180]+0)/(256+128);c3 = ([120 230 180]+128)/(256+128);patch([0 1 1 0],[0 0 1 1],c1);patch([0 1 1 1-e 1-e 0+e],[0 0 1 1-e 0+e 0+e],c2);patch([0 0 1 1-e 0+e 0+e],[0 1 1 1-e 1-e 0+e],c3);plot([0 1 1 0 0],[0 0 1 1 0]);
  Delays 1 : Subsystem
      mask Display: e = 0.06;c1 = [120 150 230]/255;c2 = ([120 150 230]+0)/383;c3 = ([120 150 230]+128)/383;patch([0 1 1 0],[0 0 1 1],c1);patch([0 1 1 1-e 1-e 0+e],[0 0 1 1-e 0+e 0+e],c2);patch([0 0 1 1-e 0+e 0+e],[0 1 1 1-e 1-e 0+e],c3);plot([0 1 1 0 0],[0 0 1 1 0]);disp('0');
  "LW{7,6}" : Subsystem
      mask Display: e = 0.06;c1 = [120 150 230]/255;c2 = ([120 150 230]+0)/383;c3 = ([120 150 230]+128)/383;patch([0 1 1 0],[0 0 1 1],c1);patch([0 1 1 1-e 1-e 0+e],[0 0 1 1-e 0+e 0+e],c2);patch([0 0 1 1-e 0+e 0+e],[0 1 1 1-e 1-e 0+e],c3);plot([0 1 1 0 0],[0 0 1 1 0]);disp('W');
  "b{7}" : Constant  Value=[-0.0204720000000000006135092434078615042380988597869873046875;-0.019676800000000001233946278489383985288441181182861328125;0.0182523999999999984866771995939416228793561458587646484375;-0.018324500000000000510258502117721945978701114654541015625;0.0187023000000000015619061599636552273295819759368896484375]
      mask Display: e = 0.06;c1 = [120 150 230]/255;c2 = ([120 150 230]+0)/383;c3 = ([120 150 230]+128)/383;patch([0 1 1 0],[0 0 1 1],c1);patch([0 1 1 1-e 1-e 0+e],[0 0 1 1-e 0+e 0+e],c2);patch([0 0 1 1-e 0+e 0+e],[0 1 1 1-e 1-e 0+e],c3);plot([0 1 1 0 0],[0 0 1 1 0]);disp('b');
  netsum : Sum  Inputs=2  SaturateOnIntegerOverflow=on  InputSameDT=on  OutDataTypeStr=Inherit: Same as first input
      mask Display: e = 0.06;c1 = [120 150 230]/255;c2 = ([120 150 230]+0)/383;c3 = ([120 150 230]+128)/383;patch([0 1 1 0],[0 0 1 1],c1);patch([0 1 1 1-e 1-e 0+e],[0 0 1 1-e 0+e 0+e],c2);patch([0 0 1 1-e 0+e 0+e],[0 1 1 1-e 1-e 0+e],c3);plot([0 1 1 0 0],[0 0 1 1 0]);disp('+');
  purelin : neural/Transfer Functions/purelin
      settings: SourceProductName=Deep Learning Toolbox
  "a{7}" : Outport  InitialOutput=[0;0;0;0;0]
      mask Display: e = 0.06;c1 = [120 230 180]/255;c2 = ([120 230 180]+0)/(256+128);c3 = ([120 230 180]+128)/(256+128);patch([0 1 1 0],[0 0 1 1],c1);patch([0 1 1 1-e 1-e 0+e],[0 0 1 1-e 0+e 0+e],c2);patch([0 0 1 1-e 0+e 0+e],[0 1 1 1-e 1-e 0+e],c3);plot([0 1 1 0 0],[0 0 1 1 0]);
  wires:
  purelin/1 -> "a{7}"/1
  netsum/1 -> purelin/1
  "b{7}"/1 -> netsum/2
  "LW{7,6}"/1("lz{7,6}") -> netsum/1
  Delays 1/1("pd{7,6}") -> "LW{7,6}"/1("ad{7,6}")
  "a{6} "/1 -> Delays 1/1("p{7}")
